simulink model "wind1000_BUS_Original"  (R2024a)
settings: Solver=ode45  StartTime=parSimulacio.ti  StopTime=40  MaxStep=parSimulacio.pas  RelTol=1e-6  AbsTol=1e-6  EnableMultiTasking=on
model workspace (MAT data in the model file):
  tt_vw : Simulink.Parameter (value not decoded)
values from neighbouring files (symbols this model uses):
  L = L'    [parameters_windFarm2.m]
  nu = size(S, 1)    [parameters_windFarm2.m]
undefined symbols (not defined in the file or in its folder): parControl, parConvertidor, parGenerador, parGrid, parMecanica, parSimulacio, S, w0, windspeed
(part 8 of 218)

== system wind1000_BUS_Original/Subsystem_200/Subsystem/String8/WT9 ==
  V_in : Inport
  I_in : Inport  Port=2
  Add1 : Sum  Inputs=+-
  C_cable : Subsystem
  C_filter : Subsystem
  Cable line : Subsystem
  Gain : Gain  Gain=1/parGrid.WTstring.Rparallel_cable
  Transformer-complex : Subsystem
  WFG : Subsystem
  V_out : Outport
  I_out : Outport  Port=2
  wires:
  I_in/1 -> C_cable/2(I_in)
  Gain/1 -> Add1/2
  Add1/1 -> C_cable/1(It2)
  Transformer-complex/2(It2) -> Add1/1
  V_in/1 -> Cable line/1(V)
  Transformer-complex/1(It1) -> C_filter/2(It1)
  Cable line/1(I_cable) -> C_cable/3(I_cable), I_out/1
  C_filter/1(Vc_filter_qd) -> Transformer-complex/1(Vc_filter_qd), WFG/1(vcqd)
  WFG/1(ilqd) -> C_filter/1(IWF)
  C_cable/1(Vc_cable) -> Cable line/2(Vc_cable), V_out/1, Transformer-complex/2(Vc_cable_qd), Gain/1

== system wind1000_BUS_Original/Subsystem_200/Subsystem/String9/WT1 ==
  V_in : Inport
  I_in : Inport  Port=2
  Add1 : Sum  Inputs=+-
  C_cable : Subsystem
  C_filter : Subsystem
  Cable line : Subsystem
  Gain : Gain  Gain=1/parGrid.WTstring.Rparallel_cable
  Transformer-complex : Subsystem
  WFG : Subsystem
  V_out : Outport
  I_out : Outport  Port=2
  wires:
  I_in/1 -> C_cable/2(I_in)
  Gain/1 -> Add1/2
  Add1/1 -> C_cable/1(It2)
  Transformer-complex/2(It2) -> Add1/1
  V_in/1 -> Cable line/1(V)
  Transformer-complex/1(It1) -> C_filter/2(It1)
  Cable line/1(I_cable) -> C_cable/3(I_cable), I_out/1
  C_filter/1(Vc_filter_qd) -> WFG/1(vcqd), Transformer-complex/1(Vc_filter_qd)
  WFG/1(ilqd) -> C_filter/1(IWF)
  C_cable/1(Vc_cable) -> Cable line/2(Vc_cable), V_out/1, Transformer-complex/2(Vc_cable_qd), Gain/1

== system wind1000_BUS_Original/Subsystem_200/Subsystem/String9/WT10 ==
  V_in : Inport
  I_in : Inport  Port=2
  Add1 : Sum  Inputs=+-
  C_cable : Subsystem
  C_filter : Subsystem
  Cable line : Subsystem
  Gain : Gain  Gain=1/parGrid.WTstring.Rparallel_cable
  Transformer-complex : Subsystem
  WFG : Subsystem
  V_out : Outport
  I_out : Outport  Port=2
  wires:
  C_cable/1(Vc_cable) -> Gain/1, Transformer-complex/2(Vc_cable_qd), V_out/1, Cable line/2(Vc_cable)
  WFG/1(ilqd) -> C_filter/1(IWF)
  C_filter/1(Vc_filter_qd) -> Transformer-complex/1(Vc_filter_qd), WFG/1(vcqd)
  Cable line/1(I_cable) -> I_out/1, C_cable/3(I_cable)
  Transformer-complex/1(It1) -> C_filter/2(It1)
  V_in/1 -> Cable line/1(V)
  Transformer-complex/2(It2) -> Add1/1
  Add1/1 -> C_cable/1(It2)
  Gain/1 -> Add1/2
  I_in/1 -> C_cable/2(I_in)

== system wind1000_BUS_Original/Subsystem_200/Subsystem/String9/WT2 ==
  V_in : Inport
  I_in : Inport  Port=2
  Add1 : Sum  Inputs=+-
  C_cable : Subsystem
  C_filter : Subsystem
  Cable line : Subsystem
  Gain : Gain  Gain=1/parGrid.WTstring.Rparallel_cable
  Transformer-complex : Subsystem
  WFG : Subsystem
  V_out : Outport
  I_out : Outport  Port=2
  wires:
  C_cable/1(Vc_cable) -> Gain/1, Transformer-complex/2(Vc_cable_qd), V_out/1, Cable line/2(Vc_cable)
  WFG/1(ilqd) -> C_filter/1(IWF)
  C_filter/1(Vc_filter_qd) -> Transformer-complex/1(Vc_filter_qd), WFG/1(vcqd)
  Cable line/1(I_cable) -> I_out/1, C_cable/3(I_cable)
  Transformer-complex/1(It1) -> C_filter/2(It1)
  V_in/1 -> Cable line/1(V)
  Transformer-complex/2(It2) -> Add1/1
  Add1/1 -> C_cable/1(It2)
  Gain/1 -> Add1/2
  I_in/1 -> C_cable/2(I_in)

== system wind1000_BUS_Original/Subsystem_200/Subsystem/String9/WT3 ==
  V_in : Inport
  I_in : Inport  Port=2
  Add1 : Sum  Inputs=+-
  C_cable : Subsystem
  C_filter : Subsystem
  Cable line : Subsystem
  Gain : Gain  Gain=1/parGrid.WTstring.Rparallel_cable
  Transformer-complex : Subsystem
  WFG : Subsystem
  V_out : Outport
  I_out : Outport  Port=2
  wires:
  I_in/1 -> C_cable/2(I_in)
  Gain/1 -> Add1/2
  Add1/1 -> C_cable/1(It2)
  Transformer-complex/2(It2) -> Add1/1
  V_in/1 -> Cable line/1(V)
  Transformer-complex/1(It1) -> C_filter/2(It1)
  Cable line/1(I_cable) -> C_cable/3(I_cable), I_out/1
  C_filter/1(Vc_filter_qd) -> Transformer-complex/1(Vc_filter_qd), WFG/1(vcqd)
  WFG/1(ilqd) -> C_filter/1(IWF)
  C_cable/1(Vc_cable) -> Cable line/2(Vc_cable), V_out/1, Transformer-complex/2(Vc_cable_qd), Gain/1

== system wind1000_BUS_Original/Subsystem_200/Subsystem/String9/WT4 ==
  V_in : Inport
  I_in : Inport  Port=2
  Add1 : Sum  Inputs=+-
  C_cable : Subsystem
  C_filter : Subsystem
  Cable line : Subsystem
  Gain : Gain  Gain=1/parGrid.WTstring.Rparallel_cable
  Transformer-complex : Subsystem
  WFG : Subsystem
  V_out : Outport
  I_out : Outport  Port=2
  wires:
  C_cable/1(Vc_cable) -> Gain/1, Transformer-complex/2(Vc_cable_qd), V_out/1, Cable line/2(Vc_cable)
  WFG/1(ilqd) -> C_filter/1(IWF)
  C_filter/1(Vc_filter_qd) -> Transformer-complex/1(Vc_filter_qd), WFG/1(vcqd)
  Cable line/1(I_cable) -> I_out/1, C_cable/3(I_cable)
  Transformer-complex/1(It1) -> C_filter/2(It1)
  V_in/1 -> Cable line/1(V)
  Transformer-complex/2(It2) -> Add1/1
  Add1/1 -> C_cable/1(It2)
  Gain/1 -> Add1/2
  I_in/1 -> C_cable/2(I_in)

== system wind1000_BUS_Original/Subsystem_200/Subsystem/String9/WT5 ==
  V_in : Inport
  I_in : Inport  Port=2
  Add1 : Sum  Inputs=+-
  C_cable : Subsystem
  C_filter : Subsystem
  Cable line : Subsystem
  Gain : Gain  Gain=1/parGrid.WTstring.Rparallel_cable
  Transformer-complex : Subsystem
  WFG : Subsystem
  V_out : Outport
  I_out : Outport  Port=2
  wires:
  I_in/1 -> C_cable/2(I_in)
  Gain/1 -> Add1/2
  Add1/1 -> C_cable/1(It2)
  Transformer-complex/2(It2) -> Add1/1
  V_in/1 -> Cable line/1(V)
  Transformer-complex/1(It1) -> C_filter/2(It1)
  Cable line/1(I_cable) -> C_cable/3(I_cable), I_out/1
  C_filter/1(Vc_filter_qd) -> Transformer-complex/1(Vc_filter_qd), WFG/1(vcqd)
  WFG/1(ilqd) -> C_filter/1(IWF)
  C_cable/1(Vc_cable) -> Cable line/2(Vc_cable), V_out/1, Transformer-complex/2(Vc_cable_qd), Gain/1

== system wind1000_BUS_Original/Subsystem_200/Subsystem/String9/WT6 ==
  V_in : Inport
  I_in : Inport  Port=2
  Add1 : Sum  Inputs=+-
  C_cable : Subsystem
  C_filter : Subsystem
  Cable line : Subsystem
  Gain : Gain  Gain=1/parGrid.WTstring.Rparallel_cable
  Transformer-complex : Subsystem
  WFG : Subsystem
  V_out : Outport
  I_out : Outport  Port=2
  wires:
  C_cable/1(Vc_cable) -> Gain/1, Transformer-complex/2(Vc_cable_qd), V_out/1, Cable line/2(Vc_cable)
  WFG/1(ilqd) -> C_filter/1(IWF)
  C_filter/1(Vc_filter_qd) -> Transformer-complex/1(Vc_filter_qd), WFG/1(vcqd)
  Cable line/1(I_cable) -> I_out/1, C_cable/3(I_cable)
  Transformer-complex/1(It1) -> C_filter/2(It1)
  V_in/1 -> Cable line/1(V)
  Transformer-complex/2(It2) -> Add1/1
  Add1/1 -> C_cable/1(It2)
  Gain/1 -> Add1/2
  I_in/1 -> C_cable/2(I_in)

== system wind1000_BUS_Original/Subsystem_200/Subsystem/String9/WT7 ==
  V_in : Inport
  I_in : Inport  Port=2
  Add1 : Sum  Inputs=+-
  C_cable : Subsystem
  C_filter : Subsystem
  Cable line : Subsystem
  Gain : Gain  Gain=1/parGrid.WTstring.Rparallel_cable
  Transformer-complex : Subsystem
  WFG : Subsystem
  V_out : Outport
  I_out : Outport  Port=2
  wires:
  I_in/1 -> C_cable/2(I_in)
  Gain/1 -> Add1/2
  Add1/1 -> C_cable/1(It2)
  Transformer-complex/2(It2) -> Add1/1
  V_in/1 -> Cable line/1(V)
  Transformer-complex/1(It1) -> C_filter/2(It1)
  Cable line/1(I_cable) -> C_cable/3(I_cable), I_out/1
  C_filter/1(Vc_filter_qd) -> Transformer-complex/1(Vc_filter_qd), WFG/1(vcqd)
  WFG/1(ilqd) -> C_filter/1(IWF)
  C_cable/1(Vc_cable) -> Cable line/2(Vc_cable), V_out/1, Transformer-complex/2(Vc_cable_qd), Gain/1

== system wind1000_BUS_Original/Subsystem_200/Subsystem/String9/WT8 ==
  V_in : Inport
  I_in : Inport  Port=2
  Add1 : Sum  Inputs=+-
  C_cable : Subsystem
  C_filter : Subsystem
  Cable line : Subsystem
  Gain : Gain  Gain=1/parGrid.WTstring.Rparallel_cable
  Transformer-complex : Subsystem
  WFG : Subsystem
  V_out : Outport
  I_out : Outport  Port=2
  wires:
  C_cable/1(Vc_cable) -> Gain/1, Transformer-complex/2(Vc_cable_qd), V_out/1, Cable line/2(Vc_cable)
  WFG/1(ilqd) -> C_filter/1(IWF)
  C_filter/1(Vc_filter_qd) -> Transformer-complex/1(Vc_filter_qd), WFG/1(vcqd)
  Cable line/1(I_cable) -> I_out/1, C_cable/3(I_cable)
  Transformer-complex/1(It1) -> C_filter/2(It1)
  V_in/1 -> Cable line/1(V)
  Transformer-complex/2(It2) -> Add1/1
  Add1/1 -> C_cable/1(It2)
  Gain/1 -> Add1/2
  I_in/1 -> C_cable/2(I_in)

== system wind1000_BUS_Original/Subsystem_200/Subsystem/String9/WT9 ==
  V_in : Inport
  I_in : Inport  Port=2
  Add1 : Sum  Inputs=+-
  C_cable : Subsystem
  C_filter : Subsystem
  Cable line : Subsystem
  Gain : Gain  Gain=1/parGrid.WTstring.Rparallel_cable
  Transformer-complex : Subsystem
  WFG : Subsystem
  V_out : Outport
  I_out : Outport  Port=2
  wires:
  I_in/1 -> C_cable/2(I_in)
  Gain/1 -> Add1/2
  Add1/1 -> C_cable/1(It2)
  Transformer-complex/2(It2) -> Add1/1
  V_in/1 -> Cable line/1(V)
  Transformer-complex/1(It1) -> C_filter/2(It1)
  Cable line/1(I_cable) -> C_cable/3(I_cable), I_out/1
  C_filter/1(Vc_filter_qd) -> Transformer-complex/1(Vc_filter_qd), WFG/1(vcqd)
  WFG/1(ilqd) -> C_filter/1(IWF)
  C_cable/1(Vc_cable) -> Cable line/2(Vc_cable), V_out/1, Transformer-complex/2(Vc_cable_qd), Gain/1

== system wind1000_BUS_Original/Subsystem/Subsystem_1/3phase2dq/Font trifasica asimetrica1/Park ==
  theta_r : Inport
  Constant4 : Constant  Value=2*pi/3
  Gain7 : Gain  Gain=2/3
  Matrix Concatenate1 : Concatenate  NumInputs=3  Mode=Multidimensional array  ConcatenateDimension=2
  Matrix Concatenate2 : Concatenate  Mode=Multidimensional array  ConcatenateDimension=2
  Mux2 : Mux  Inputs=2
  Mux3 : Mux  Inputs=2
  Mux4 : Mux  Inputs=2
  Mux5 : Mux  Inputs=3
  Mux6 : Mux  Inputs=3
  Sum1 : Sum  Inputs=|++
  Sum2 : Sum  Inputs=|+-
  Trigonometric Function : Trigonometry  Operator=cos
  Trigonometric Function1 : Trigonometry
  Trigonometric Function2 : Trigonometry  Operator=cos
  Trigonometric Function3 : Trigonometry
  Trigonometric Function4 : Trigonometry  Operator=cos
  Trigonometric Function5 : Trigonometry
  Tthetar : Outport
  nTthetar : Outport  Port=2
  wires:
  theta_r/1 -> Sum1/1, Sum2/1, Trigonometric Function/1, Trigonometric Function1/1
  Sum2/1 -> Trigonometric Function2/1, Trigonometric Function3/1
  Sum1/1 -> Trigonometric Function4/1, Trigonometric Function5/1
  Constant4/1 -> Sum2/2, Sum1/2
  Trigonometric Function/1 -> Mux2/1, Mux5/1
  Trigonometric Function1/1 -> Mux2/2, Mux6/1
  Trigonometric Function2/1 -> Mux3/1, Mux5/2
  Trigonometric Function3/1 -> Mux3/2, Mux6/2
  Trigonometric Function4/1 -> Mux4/1, Mux5/3
  Trigonometric Function5/1 -> Mux4/2, Mux6/3
  Mux2/1 -> Matrix Concatenate1/1
  Mux3/1 -> Matrix Concatenate1/2
  Mux4/1 -> Matrix Concatenate1/3
  Matrix Concatenate1/1 -> Gain7/1
  Mux5/1 -> Matrix Concatenate2/1
  Mux6/1 -> Matrix Concatenate2/2
  Gain7/1 -> Tthetar/1
  Matrix Concatenate2/1 -> nTthetar/1

== system wind1000_BUS_Original/Subsystem/Subsystem_1/Subsystem/String1/WT1 ==
  V_in : Inport
  I_in : Inport  Port=2
  Add1 : Sum  Inputs=+-
  C_cable : Subsystem
  C_filter : Subsystem
  Cable line : Subsystem
  Gain : Gain  Gain=1/parGrid.WTstring.Rparallel_cable
  Transformer-complex : Subsystem
  WFG : Subsystem
  V_out : Outport
  I_out : Outport  Port=2
  wires:
  C_cable/1(Vc_cable) -> Gain/1, Transformer-complex/2(Vc_cable_qd), V_out/1, Cable line/2(Vc_cable)
  WFG/1(ilqd) -> C_filter/1(IWF)
  C_filter/1(Vc_filter_qd) -> Transformer-complex/1(Vc_filter_qd), WFG/1(vcqd)
  Cable line/1(I_cable) -> I_out/1, C_cable/3(I_cable)
  Transformer-complex/1(It1) -> C_filter/2(It1)
  V_in/1 -> Cable line/1(V)
  Transformer-complex/2(It2) -> Add1/1
  Add1/1 -> C_cable/1(It2)
  Gain/1 -> Add1/2
  I_in/1 -> C_cable/2(I_in)

== system wind1000_BUS_Original/Subsystem/Subsystem_1/Subsystem/String1/WT10 ==
  V_in : Inport
  I_in : Inport  Port=2
  Add1 : Sum  Inputs=+-
  C_cable : Subsystem
  C_filter : Subsystem
  Cable line : Subsystem
  Gain : Gain  Gain=1/parGrid.WTstring.Rparallel_cable
  Transformer-complex : Subsystem
  WFG : Subsystem
  V_out : Outport
  I_out : Outport  Port=2
  wires:
  I_in/1 -> C_cable/2(I_in)
  Gain/1 -> Add1/2
  Add1/1 -> C_cable/1(It2)
  Transformer-complex/2(It2) -> Add1/1
  V_in/1 -> Cable line/1(V)
  Transformer-complex/1(It1) -> C_filter/2(It1)
  Cable line/1(I_cable) -> C_cable/3(I_cable), I_out/1
  C_filter/1(Vc_filter_qd) -> WFG/1(vcqd), Transformer-complex/1(Vc_filter_qd)
  WFG/1(ilqd) -> C_filter/1(IWF)
  C_cable/1(Vc_cable) -> Cable line/2(Vc_cable), V_out/1, Transformer-complex/2(Vc_cable_qd), Gain/1

== system wind1000_BUS_Original/Subsystem/Subsystem_1/Subsystem/String1/WT2 ==
  V_in : Inport
  I_in : Inport  Port=2
  Add1 : Sum  Inputs=+-
  C_cable : Subsystem
  C_filter : Subsystem
  Cable line : Subsystem
  Gain : Gain  Gain=1/parGrid.WTstring.Rparallel_cable
  Transformer-complex : Subsystem
  WFG : Subsystem
  V_out : Outport
  I_out : Outport  Port=2
  wires:
  I_in/1 -> C_cable/2(I_in)
  Gain/1 -> Add1/2
  Add1/1 -> C_cable/1(It2)
  Transformer-complex/2(It2) -> Add1/1
  V_in/1 -> Cable line/1(V)
  Transformer-complex/1(It1) -> C_filter/2(It1)
  Cable line/1(I_cable) -> C_cable/3(I_cable), I_out/1
  C_filter/1(Vc_filter_qd) -> WFG/1(vcqd), Transformer-complex/1(Vc_filter_qd)
  WFG/1(ilqd) -> C_filter/1(IWF)
  C_cable/1(Vc_cable) -> Cable line/2(Vc_cable), V_out/1, Transformer-complex/2(Vc_cable_qd), Gain/1

== system wind1000_BUS_Original/Subsystem/Subsystem_1/Subsystem/String1/WT3 ==
  V_in : Inport
  I_in : Inport  Port=2
  Add1 : Sum  Inputs=+-
  C_cable : Subsystem
  C_filter : Subsystem
  Cable line : Subsystem
  Gain : Gain  Gain=1/parGrid.WTstring.Rparallel_cable
  Transformer-complex : Subsystem
  WFG : Subsystem
  V_out : Outport
  I_out : Outport  Port=2
  wires:
  C_cable/1(Vc_cable) -> Gain/1, Transformer-complex/2(Vc_cable_qd), V_out/1, Cable line/2(Vc_cable)
  WFG/1(ilqd) -> C_filter/1(IWF)
  C_filter/1(Vc_filter_qd) -> WFG/1(vcqd), Transformer-complex/1(Vc_filter_qd)
  Cable line/1(I_cable) -> I_out/1, C_cable/3(I_cable)
  Transformer-complex/1(It1) -> C_filter/2(It1)
  V_in/1 -> Cable line/1(V)
  Transformer-complex/2(It2) -> Add1/1
  Add1/1 -> C_cable/1(It2)
  Gain/1 -> Add1/2
  I_in/1 -> C_cable/2(I_in)

== system wind1000_BUS_Original/Subsystem/Subsystem_1/Subsystem/String1/WT4 ==
  V_in : Inport
  I_in : Inport  Port=2
  Add1 : Sum  Inputs=+-
  C_cable : Subsystem
  C_filter : Subsystem
  Cable line : Subsystem
  Gain : Gain  Gain=1/parGrid.WTstring.Rparallel_cable
  Transformer-complex : Subsystem
  WFG : Subsystem
  V_out : Outport
  I_out : Outport  Port=2
  wires:
  I_in/1 -> C_cable/2(I_in)
  Gain/1 -> Add1/2
  Add1/1 -> C_cable/1(It2)
  Transformer-complex/2(It2) -> Add1/1
  V_in/1 -> Cable line/1(V)
  Transformer-complex/1(It1) -> C_filter/2(It1)
  Cable line/1(I_cable) -> C_cable/3(I_cable), I_out/1
  C_filter/1(Vc_filter_qd) -> WFG/1(vcqd), Transformer-complex/1(Vc_filter_qd)
  WFG/1(ilqd) -> C_filter/1(IWF)
  C_cable/1(Vc_cable) -> Cable line/2(Vc_cable), V_out/1, Transformer-complex/2(Vc_cable_qd), Gain/1

== system wind1000_BUS_Original/Subsystem/Subsystem_1/Subsystem/String1/WT5 ==
  V_in : Inport
  I_in : Inport  Port=2
  Add1 : Sum  Inputs=+-
  C_cable : Subsystem
  C_filter : Subsystem
  Cable line : Subsystem
  Gain : Gain  Gain=1/parGrid.WTstring.Rparallel_cable
  Transformer-complex : Subsystem
  WFG : Subsystem
  V_out : Outport
  I_out : Outport  Port=2
  wires:
  C_cable/1(Vc_cable) -> Gain/1, Transformer-complex/2(Vc_cable_qd), V_out/1, Cable line/2(Vc_cable)
  WFG/1(ilqd) -> C_filter/1(IWF)
  C_filter/1(Vc_filter_qd) -> WFG/1(vcqd), Transformer-complex/1(Vc_filter_qd)
  Cable line/1(I_cable) -> I_out/1, C_cable/3(I_cable)
  Transformer-complex/1(It1) -> C_filter/2(It1)
  V_in/1 -> Cable line/1(V)
  Transformer-complex/2(It2) -> Add1/1
  Add1/1 -> C_cable/1(It2)
  Gain/1 -> Add1/2
  I_in/1 -> C_cable/2(I_in)

== system wind1000_BUS_Original/Subsystem/Subsystem_1/Subsystem/String1/WT6 ==
  V_in : Inport
  I_in : Inport  Port=2
  Add1 : Sum  Inputs=+-
  C_cable : Subsystem
  C_filter : Subsystem
  Cable line : Subsystem
  Gain : Gain  Gain=1/parGrid.WTstring.Rparallel_cable
  Transformer-complex : Subsystem
  WFG : Subsystem
  V_out : Outport
  I_out : Outport  Port=2
  wires:
  I_in/1 -> C_cable/2(I_in)
  Gain/1 -> Add1/2
  Add1/1 -> C_cable/1(It2)
  Transformer-complex/2(It2) -> Add1/1
  V_in/1 -> Cable line/1(V)
  Transformer-complex/1(It1) -> C_filter/2(It1)
  Cable line/1(I_cable) -> C_cable/3(I_cable), I_out/1
  C_filter/1(Vc_filter_qd) -> WFG/1(vcqd), Transformer-complex/1(Vc_filter_qd)
  WFG/1(ilqd) -> C_filter/1(IWF)
  C_cable/1(Vc_cable) -> Cable line/2(Vc_cable), V_out/1, Transformer-complex/2(Vc_cable_qd), Gain/1

== system wind1000_BUS_Original/Subsystem/Subsystem_1/Subsystem/String1/WT7 ==
  V_in : Inport
  I_in : Inport  Port=2
  Add1 : Sum  Inputs=+-
  C_cable : Subsystem
  C_filter : Subsystem
  Cable line : Subsystem
  Gain : Gain  Gain=1/parGrid.WTstring.Rparallel_cable
  Transformer-complex : Subsystem
  WFG : Subsystem
  V_out : Outport
  I_out : Outport  Port=2
  wires:
  C_cable/1(Vc_cable) -> Gain/1, Transformer-complex/2(Vc_cable_qd), V_out/1, Cable line/2(Vc_cable)
  WFG/1(ilqd) -> C_filter/1(IWF)
  C_filter/1(Vc_filter_qd) -> WFG/1(vcqd), Transformer-complex/1(Vc_filter_qd)
  Cable line/1(I_cable) -> I_out/1, C_cable/3(I_cable)
  Transformer-complex/1(It1) -> C_filter/2(It1)
  V_in/1 -> Cable line/1(V)
  Transformer-complex/2(It2) -> Add1/1
  Add1/1 -> C_cable/1(It2)
  Gain/1 -> Add1/2
  I_in/1 -> C_cable/2(I_in)

== system wind1000_BUS_Original/Subsystem/Subsystem_1/Subsystem/String1/WT8 ==
  V_in : Inport
  I_in : Inport  Port=2
  Add1 : Sum  Inputs=+-
  C_cable : Subsystem
  C_filter : Subsystem
  Cable line : Subsystem
  Gain : Gain  Gain=1/parGrid.WTstring.Rparallel_cable
  Transformer-complex : Subsystem
  WFG : Subsystem
  V_out : Outport
  I_out : Outport  Port=2
  wires:
  I_in/1 -> C_cable/2(I_in)
  Gain/1 -> Add1/2
  Add1/1 -> C_cable/1(It2)
  Transformer-complex/2(It2) -> Add1/1
  V_in/1 -> Cable line/1(V)
  Transformer-complex/1(It1) -> C_filter/2(It1)
  Cable line/1(I_cable) -> C_cable/3(I_cable), I_out/1
  C_filter/1(Vc_filter_qd) -> WFG/1(vcqd), Transformer-complex/1(Vc_filter_qd)
  WFG/1(ilqd) -> C_filter/1(IWF)
  C_cable/1(Vc_cable) -> Cable line/2(Vc_cable), V_out/1, Transformer-complex/2(Vc_cable_qd), Gain/1

== system wind1000_BUS_Original/Subsystem/Subsystem_1/Subsystem/String1/WT9 ==
  V_in : Inport
  I_in : Inport  Port=2
  Add1 : Sum  Inputs=+-
  C_cable : Subsystem
  C_filter : Subsystem
  Cable line : Subsystem
  Gain : Gain  Gain=1/parGrid.WTstring.Rparallel_cable
  Transformer-complex : Subsystem
  WFG : Subsystem
  V_out : Outport
  I_out : Outport  Port=2
  wires:
  C_cable/1(Vc_cable) -> Gain/1, Transformer-complex/2(Vc_cable_qd), V_out/1, Cable line/2(Vc_cable)
  WFG/1(ilqd) -> C_filter/1(IWF)
  C_filter/1(Vc_filter_qd) -> WFG/1(vcqd), Transformer-complex/1(Vc_filter_qd)
  Cable line/1(I_cable) -> I_out/1, C_cable/3(I_cable)
  Transformer-complex/1(It1) -> C_filter/2(It1)
  V_in/1 -> Cable line/1(V)
  Transformer-complex/2(It2) -> Add1/1
  Add1/1 -> C_cable/1(It2)
  Gain/1 -> Add1/2
  I_in/1 -> C_cable/2(I_in)

== system wind1000_BUS_Original/Subsystem/Subsystem_1/Subsystem/String2/WT1 ==
  V_in : Inport
  I_in : Inport  Port=2
  Add1 : Sum  Inputs=+-
  C_cable : Subsystem
  C_filter : Subsystem
  Cable line : Subsystem
  Gain : Gain  Gain=1/parGrid.WTstring.Rparallel_cable
  Transformer-complex : Subsystem
  WFG : Subsystem
  V_out : Outport
  I_out : Outport  Port=2
  wires:
  I_in/1 -> C_cable/2(I_in)
  Gain/1 -> Add1/2
  Add1/1 -> C_cable/1(It2)
  Transformer-complex/2(It2) -> Add1/1
  V_in/1 -> Cable line/1(V)
  Transformer-complex/1(It1) -> C_filter/2(It1)
  Cable line/1(I_cable) -> C_cable/3(I_cable), I_out/1
  C_filter/1(Vc_filter_qd) -> WFG/1(vcqd), Transformer-complex/1(Vc_filter_qd)
  WFG/1(ilqd) -> C_filter/1(IWF)
  C_cable/1(Vc_cable) -> Cable line/2(Vc_cable), V_out/1, Transformer-complex/2(Vc_cable_qd), Gain/1

== system wind1000_BUS_Original/Subsystem/Subsystem_1/Subsystem/String2/WT10 ==
  V_in : Inport
  I_in : Inport  Port=2
  Add1 : Sum  Inputs=+-
  C_cable : Subsystem
  C_filter : Subsystem
  Cable line : Subsystem
  Gain : Gain  Gain=1/parGrid.WTstring.Rparallel_cable
  Transformer-complex : Subsystem
  WFG : Subsystem
  V_out : Outport
  I_out : Outport  Port=2
  wires:
  C_cable/1(Vc_cable) -> Gain/1, Transformer-complex/2(Vc_cable_qd), V_out/1, Cable line/2(Vc_cable)
  WFG/1(ilqd) -> C_filter/1(IWF)
  C_filter/1(Vc_filter_qd) -> Transformer-complex/1(Vc_filter_qd), WFG/1(vcqd)
  Cable line/1(I_cable) -> I_out/1, C_cable/3(I_cable)
  Transformer-complex/1(It1) -> C_filter/2(It1)
  V_in/1 -> Cable line/1(V)
  Transformer-complex/2(It2) -> Add1/1
  Add1/1 -> C_cable/1(It2)
  Gain/1 -> Add1/2
  I_in/1 -> C_cable/2(I_in)

== system wind1000_BUS_Original/Subsystem/Subsystem_1/Subsystem/String2/WT2 ==
  V_in : Inport
  I_in : Inport  Port=2
  Add1 : Sum  Inputs=+-
  C_cable : Subsystem
  C_filter : Subsystem
  Cable line : Subsystem
  Gain : Gain  Gain=1/parGrid.WTstring.Rparallel_cable
  Transformer-complex : Subsystem
  WFG : Subsystem
  V_out : Outport
  I_out : Outport  Port=2
  wires:
  C_cable/1(Vc_cable) -> Gain/1, Transformer-complex/2(Vc_cable_qd), V_out/1, Cable line/2(Vc_cable)
  WFG/1(ilqd) -> C_filter/1(IWF)
  C_filter/1(Vc_filter_qd) -> Transformer-complex/1(Vc_filter_qd), WFG/1(vcqd)
  Cable line/1(I_cable) -> I_out/1, C_cable/3(I_cable)
  Transformer-complex/1(It1) -> C_filter/2(It1)
  V_in/1 -> Cable line/1(V)
  Transformer-complex/2(It2) -> Add1/1
  Add1/1 -> C_cable/1(It2)
  Gain/1 -> Add1/2
  I_in/1 -> C_cable/2(I_in)

== system wind1000_BUS_Original/Subsystem/Subsystem_1/Subsystem/String2/WT3 ==
  V_in : Inport
  I_in : Inport  Port=2
  Add1 : Sum  Inputs=+-
  C_cable : Subsystem
  C_filter : Subsystem
  Cable line : Subsystem
  Gain : Gain  Gain=1/parGrid.WTstring.Rparallel_cable
  Transformer-complex : Subsystem
  WFG : Subsystem
  V_out : Outport
  I_out : Outport  Port=2
  wires:
  I_in/1 -> C_cable/2(I_in)
  Gain/1 -> Add1/2
  Add1/1 -> C_cable/1(It2)
  Transformer-complex/2(It2) -> Add1/1
  V_in/1 -> Cable line/1(V)
  Transformer-complex/1(It1) -> C_filter/2(It1)
  Cable line/1(I_cable) -> C_cable/3(I_cable), I_out/1
  C_filter/1(Vc_filter_qd) -> Transformer-complex/1(Vc_filter_qd), WFG/1(vcqd)
  WFG/1(ilqd) -> C_filter/1(IWF)
  C_cable/1(Vc_cable) -> Cable line/2(Vc_cable), V_out/1, Transformer-complex/2(Vc_cable_qd), Gain/1

== system wind1000_BUS_Original/Subsystem/Subsystem_1/Subsystem/String2/WT4 ==
  V_in : Inport
  I_in : Inport  Port=2
  Add1 : Sum  Inputs=+-
  C_cable : Subsystem
  C_filter : Subsystem
  Cable line : Subsystem
  Gain : Gain  Gain=1/parGrid.WTstring.Rparallel_cable
  Transformer-complex : Subsystem
  WFG : Subsystem
  V_out : Outport
  I_out : Outport  Port=2
  wires:
  C_cable/1(Vc_cable) -> Gain/1, Transformer-complex/2(Vc_cable_qd), V_out/1, Cable line/2(Vc_cable)
  WFG/1(ilqd) -> C_filter/1(IWF)
  C_filter/1(Vc_filter_qd) -> Transformer-complex/1(Vc_filter_qd), WFG/1(vcqd)
  Cable line/1(I_cable) -> I_out/1, C_cable/3(I_cable)
  Transformer-complex/1(It1) -> C_filter/2(It1)
  V_in/1 -> Cable line/1(V)
  Transformer-complex/2(It2) -> Add1/1
  Add1/1 -> C_cable/1(It2)
  Gain/1 -> Add1/2
  I_in/1 -> C_cable/2(I_in)

== system wind1000_BUS_Original/Subsystem/Subsystem_1/Subsystem/String2/WT5 ==
  V_in : Inport
  I_in : Inport  Port=2
  Add1 : Sum  Inputs=+-
  C_cable : Subsystem
  C_filter : Subsystem
  Cable line : Subsystem
  Gain : Gain  Gain=1/parGrid.WTstring.Rparallel_cable
  Transformer-complex : Subsystem
  WFG : Subsystem
  V_out : Outport
  I_out : Outport  Port=2
  wires:
  I_in/1 -> C_cable/2(I_in)
  Gain/1 -> Add1/2
  Add1/1 -> C_cable/1(It2)
  Transformer-complex/2(It2) -> Add1/1
  V_in/1 -> Cable line/1(V)
  Transformer-complex/1(It1) -> C_filter/2(It1)
  Cable line/1(I_cable) -> C_cable/3(I_cable), I_out/1
  C_filter/1(Vc_filter_qd) -> Transformer-complex/1(Vc_filter_qd), WFG/1(vcqd)
  WFG/1(ilqd) -> C_filter/1(IWF)
  C_cable/1(Vc_cable) -> Cable line/2(Vc_cable), V_out/1, Transformer-complex/2(Vc_cable_qd), Gain/1

== system wind1000_BUS_Original/Subsystem/Subsystem_1/Subsystem/String2/WT6 ==
  V_in : Inport
  I_in : Inport  Port=2
  Add1 : Sum  Inputs=+-
  C_cable : Subsystem
  C_filter : Subsystem
  Cable line : Subsystem
  Gain : Gain  Gain=1/parGrid.WTstring.Rparallel_cable
  Transformer-complex : Subsystem
  WFG : Subsystem
  V_out : Outport
  I_out : Outport  Port=2
  wires:
  C_cable/1(Vc_cable) -> Gain/1, Transformer-complex/2(Vc_cable_qd), V_out/1, Cable line/2(Vc_cable)
  WFG/1(ilqd) -> C_filter/1(IWF)
  C_filter/1(Vc_filter_qd) -> Transformer-complex/1(Vc_filter_qd), WFG/1(vcqd)
  Cable line/1(I_cable) -> I_out/1, C_cable/3(I_cable)
  Transformer-complex/1(It1) -> C_filter/2(It1)
  V_in/1 -> Cable line/1(V)
  Transformer-complex/2(It2) -> Add1/1
  Add1/1 -> C_cable/1(It2)
  Gain/1 -> Add1/2
  I_in/1 -> C_cable/2(I_in)

== system wind1000_BUS_Original/Subsystem/Subsystem_1/Subsystem/String2/WT7 ==
  V_in : Inport
  I_in : Inport  Port=2
  Add1 : Sum  Inputs=+-
  C_cable : Subsystem
  C_filter : Subsystem
  Cable line : Subsystem
  Gain : Gain  Gain=1/parGrid.WTstring.Rparallel_cable
  Transformer-complex : Subsystem
  WFG : Subsystem
  V_out : Outport
  I_out : Outport  Port=2
  wires:
  I_in/1 -> C_cable/2(I_in)
  Gain/1 -> Add1/2
  Add1/1 -> C_cable/1(It2)
  Transformer-complex/2(It2) -> Add1/1
  V_in/1 -> Cable line/1(V)
  Transformer-complex/1(It1) -> C_filter/2(It1)
  Cable line/1(I_cable) -> C_cable/3(I_cable), I_out/1
  C_filter/1(Vc_filter_qd) -> Transformer-complex/1(Vc_filter_qd), WFG/1(vcqd)
  WFG/1(ilqd) -> C_filter/1(IWF)
  C_cable/1(Vc_cable) -> Cable line/2(Vc_cable), V_out/1, Transformer-complex/2(Vc_cable_qd), Gain/1

== system wind1000_BUS_Original/Subsystem/Subsystem_1/Subsystem/String2/WT8 ==
  V_in : Inport
  I_in : Inport  Port=2
  Add1 : Sum  Inputs=+-
  C_cable : Subsystem
  C_filter : Subsystem
  Cable line : Subsystem
  Gain : Gain  Gain=1/parGrid.WTstring.Rparallel_cable
  Transformer-complex : Subsystem
  WFG : Subsystem
  V_out : Outport
  I_out : Outport  Port=2
  wires:
  C_cable/1(Vc_cable) -> Gain/1, Transformer-complex/2(Vc_cable_qd), V_out/1, Cable line/2(Vc_cable)
  WFG/1(ilqd) -> C_filter/1(IWF)
  C_filter/1(Vc_filter_qd) -> Transformer-complex/1(Vc_filter_qd), WFG/1(vcqd)
  Cable line/1(I_cable) -> I_out/1, C_cable/3(I_cable)
  Transformer-complex/1(It1) -> C_filter/2(It1)
  V_in/1 -> Cable line/1(V)
  Transformer-complex/2(It2) -> Add1/1
  Add1/1 -> C_cable/1(It2)
  Gain/1 -> Add1/2
  I_in/1 -> C_cable/2(I_in)

== system wind1000_BUS_Original/Subsystem/Subsystem_1/Subsystem/String2/WT9 ==
  V_in : Inport
  I_in : Inport  Port=2
  Add1 : Sum  Inputs=+-
  C_cable : Subsystem
  C_filter : Subsystem
  Cable line : Subsystem
  Gain : Gain  Gain=1/parGrid.WTstring.Rparallel_cable
  Transformer-complex : Subsystem
  WFG : Subsystem
  V_out : Outport
  I_out : Outport  Port=2
  wires:
  I_in/1 -> C_cable/2(I_in)
  Gain/1 -> Add1/2
  Add1/1 -> C_cable/1(It2)
  Transformer-complex/2(It2) -> Add1/1
  V_in/1 -> Cable line/1(V)
  Transformer-complex/1(It1) -> C_filter/2(It1)
  Cable line/1(I_cable) -> C_cable/3(I_cable), I_out/1
  C_filter/1(Vc_filter_qd) -> Transformer-complex/1(Vc_filter_qd), WFG/1(vcqd)
  WFG/1(ilqd) -> C_filter/1(IWF)
  C_cable/1(Vc_cable) -> Cable line/2(Vc_cable), V_out/1, Transformer-complex/2(Vc_cable_qd), Gain/1

== system wind1000_BUS_Original/Subsystem/Subsystem_1/Subsystem/String3/WT1 ==
  V_in : Inport
  I_in : Inport  Port=2
  Add1 : Sum  Inputs=+-
  C_cable : Subsystem
  C_filter : Subsystem
  Cable line : Subsystem
  Gain : Gain  Gain=1/parGrid.WTstring.Rparallel_cable
  Transformer-complex : Subsystem
  WFG : Subsystem
  V_out : Outport
  I_out : Outport  Port=2
  wires:
  I_in/1 -> C_cable/2(I_in)
  Gain/1 -> Add1/2
  Add1/1 -> C_cable/1(It2)
  Transformer-complex/2(It2) -> Add1/1
  V_in/1 -> Cable line/1(V)
  Transformer-complex/1(It1) -> C_filter/2(It1)
  Cable line/1(I_cable) -> C_cable/3(I_cable), I_out/1
  C_filter/1(Vc_filter_qd) -> WFG/1(vcqd), Transformer-complex/1(Vc_filter_qd)
  WFG/1(ilqd) -> C_filter/1(IWF)
  C_cable/1(Vc_cable) -> Cable line/2(Vc_cable), V_out/1, Transformer-complex/2(Vc_cable_qd), Gain/1

== system wind1000_BUS_Original/Subsystem/Subsystem_1/Subsystem/String3/WT10 ==
  V_in : Inport
  I_in : Inport  Port=2
  Add1 : Sum  Inputs=+-
  C_cable : Subsystem
  C_filter : Subsystem
  Cable line : Subsystem
  Gain : Gain  Gain=1/parGrid.WTstring.Rparallel_cable
  Transformer-complex : Subsystem
  WFG : Subsystem
  V_out : Outport
  I_out : Outport  Port=2
  wires:
  C_cable/1(Vc_cable) -> Gain/1, Transformer-complex/2(Vc_cable_qd), V_out/1, Cable line/2(Vc_cable)
  WFG/1(ilqd) -> C_filter/1(IWF)
  C_filter/1(Vc_filter_qd) -> Transformer-complex/1(Vc_filter_qd), WFG/1(vcqd)
  Cable line/1(I_cable) -> I_out/1, C_cable/3(I_cable)
  Transformer-complex/1(It1) -> C_filter/2(It1)
  V_in/1 -> Cable line/1(V)
  Transformer-complex/2(It2) -> Add1/1
  Add1/1 -> C_cable/1(It2)
  Gain/1 -> Add1/2
  I_in/1 -> C_cable/2(I_in)

== system wind1000_BUS_Original/Subsystem/Subsystem_1/Subsystem/String3/WT2 ==
  V_in : Inport
  I_in : Inport  Port=2
  Add1 : Sum  Inputs=+-
  C_cable : Subsystem
  C_filter : Subsystem
  Cable line : Subsystem
  Gain : Gain  Gain=1/parGrid.WTstring.Rparallel_cable
  Transformer-complex : Subsystem
  WFG : Subsystem
  V_out : Outport
  I_out : Outport  Port=2
  wires:
  C_cable/1(Vc_cable) -> Gain/1, Transformer-complex/2(Vc_cable_qd), V_out/1, Cable line/2(Vc_cable)
  WFG/1(ilqd) -> C_filter/1(IWF)
  C_filter/1(Vc_filter_qd) -> Transformer-complex/1(Vc_filter_qd), WFG/1(vcqd)
  Cable line/1(I_cable) -> I_out/1, C_cable/3(I_cable)
  Transformer-complex/1(It1) -> C_filter/2(It1)
  V_in/1 -> Cable line/1(V)
  Transformer-complex/2(It2) -> Add1/1
  Add1/1 -> C_cable/1(It2)
  Gain/1 -> Add1/2
  I_in/1 -> C_cable/2(I_in)

== system wind1000_BUS_Original/Subsystem/Subsystem_1/Subsystem/String3/WT3 ==
  V_in : Inport
  I_in : Inport  Port=2
  Add1 : Sum  Inputs=+-
  C_cable : Subsystem
  C_filter : Subsystem
  Cable line : Subsystem
  Gain : Gain  Gain=1/parGrid.WTstring.Rparallel_cable
  Transformer-complex : Subsystem
  WFG : Subsystem
  V_out : Outport
  I_out : Outport  Port=2
  wires:
  I_in/1 -> C_cable/2(I_in)
  Gain/1 -> Add1/2
  Add1/1 -> C_cable/1(It2)
  Transformer-complex/2(It2) -> Add1/1
  V_in/1 -> Cable line/1(V)
  Transformer-complex/1(It1) -> C_filter/2(It1)
  Cable line/1(I_cable) -> C_cable/3(I_cable), I_out/1
  C_filter/1(Vc_filter_qd) -> Transformer-complex/1(Vc_filter_qd), WFG/1(vcqd)
  WFG/1(ilqd) -> C_filter/1(IWF)
  C_cable/1(Vc_cable) -> Cable line/2(Vc_cable), V_out/1, Transformer-complex/2(Vc_cable_qd), Gain/1

== system wind1000_BUS_Original/Subsystem/Subsystem_1/Subsystem/String3/WT4 ==
  V_in : Inport
  I_in : Inport  Port=2
  Add1 : Sum  Inputs=+-
  C_cable : Subsystem
  C_filter : Subsystem
  Cable line : Subsystem
  Gain : Gain  Gain=1/parGrid.WTstring.Rparallel_cable
  Transformer-complex : Subsystem
  WFG : Subsystem
  V_out : Outport
  I_out : Outport  Port=2
  wires:
  C_cable/1(Vc_cable) -> Gain/1, Transformer-complex/2(Vc_cable_qd), V_out/1, Cable line/2(Vc_cable)
  WFG/1(ilqd) -> C_filter/1(IWF)
  C_filter/1(Vc_filter_qd) -> Transformer-complex/1(Vc_filter_qd), WFG/1(vcqd)
  Cable line/1(I_cable) -> I_out/1, C_cable/3(I_cable)
  Transformer-complex/1(It1) -> C_filter/2(It1)
  V_in/1 -> Cable line/1(V)
  Transformer-complex/2(It2) -> Add1/1
  Add1/1 -> C_cable/1(It2)
  Gain/1 -> Add1/2
  I_in/1 -> C_cable/2(I_in)

== system wind1000_BUS_Original/Subsystem/Subsystem_1/Subsystem/String3/WT5 ==
  V_in : Inport
  I_in : Inport  Port=2
  Add1 : Sum  Inputs=+-
  C_cable : Subsystem
  C_filter : Subsystem
  Cable line : Subsystem
  Gain : Gain  Gain=1/parGrid.WTstring.Rparallel_cable
  Transformer-complex : Subsystem
  WFG : Subsystem
  V_out : Outport
  I_out : Outport  Port=2
  wires:
  I_in/1 -> C_cable/2(I_in)
  Gain/1 -> Add1/2
  Add1/1 -> C_cable/1(It2)
  Transformer-complex/2(It2) -> Add1/1
  V_in/1 -> Cable line/1(V)
  Transformer-complex/1(It1) -> C_filter/2(It1)
  Cable line/1(I_cable) -> C_cable/3(I_cable), I_out/1
  C_filter/1(Vc_filter_qd) -> Transformer-complex/1(Vc_filter_qd), WFG/1(vcqd)
  WFG/1(ilqd) -> C_filter/1(IWF)
  C_cable/1(Vc_cable) -> Cable line/2(Vc_cable), V_out/1, Transformer-complex/2(Vc_cable_qd), Gain/1

== system wind1000_BUS_Original/Subsystem/Subsystem_1/Subsystem/String3/WT6 ==
  V_in : Inport
  I_in : Inport  Port=2
  Add1 : Sum  Inputs=+-
  C_cable : Subsystem
  C_filter : Subsystem
  Cable line : Subsystem
  Gain : Gain  Gain=1/parGrid.WTstring.Rparallel_cable
  Transformer-complex : Subsystem
  WFG : Subsystem
  V_out : Outport
  I_out : Outport  Port=2
  wires:
  C_cable/1(Vc_cable) -> Gain/1, Transformer-complex/2(Vc_cable_qd), V_out/1, Cable line/2(Vc_cable)
  WFG/1(ilqd) -> C_filter/1(IWF)
  C_filter/1(Vc_filter_qd) -> Transformer-complex/1(Vc_filter_qd), WFG/1(vcqd)
  Cable line/1(I_cable) -> I_out/1, C_cable/3(I_cable)
  Transformer-complex/1(It1) -> C_filter/2(It1)
  V_in/1 -> Cable line/1(V)
  Transformer-complex/2(It2) -> Add1/1
  Add1/1 -> C_cable/1(It2)
  Gain/1 -> Add1/2
  I_in/1 -> C_cable/2(I_in)

== system wind1000_BUS_Original/Subsystem/Subsystem_1/Subsystem/String3/WT7 ==
  V_in : Inport
  I_in : Inport  Port=2
  Add1 : Sum  Inputs=+-
  C_cable : Subsystem
  C_filter : Subsystem
  Cable line : Subsystem
  Gain : Gain  Gain=1/parGrid.WTstring.Rparallel_cable
  Transformer-complex : Subsystem
  WFG : Subsystem
  V_out : Outport
  I_out : Outport  Port=2
  wires:
  I_in/1 -> C_cable/2(I_in)
  Gain/1 -> Add1/2
  Add1/1 -> C_cable/1(It2)
  Transformer-complex/2(It2) -> Add1/1
  V_in/1 -> Cable line/1(V)
  Transformer-complex/1(It1) -> C_filter/2(It1)
  Cable line/1(I_cable) -> C_cable/3(I_cable), I_out/1
  C_filter/1(Vc_filter_qd) -> Transformer-complex/1(Vc_filter_qd), WFG/1(vcqd)
  WFG/1(ilqd) -> C_filter/1(IWF)
  C_cable/1(Vc_cable) -> Cable line/2(Vc_cable), V_out/1, Transformer-complex/2(Vc_cable_qd), Gain/1

== system wind1000_BUS_Original/Subsystem/Subsystem_1/Subsystem/String3/WT8 ==
  V_in : Inport
  I_in : Inport  Port=2
  Add1 : Sum  Inputs=+-
  C_cable : Subsystem
  C_filter : Subsystem
  Cable line : Subsystem
  Gain : Gain  Gain=1/parGrid.WTstring.Rparallel_cable
  Transformer-complex : Subsystem
  WFG : Subsystem
  V_out : Outport
  I_out : Outport  Port=2
  wires:
  C_cable/1(Vc_cable) -> Gain/1, Transformer-complex/2(Vc_cable_qd), V_out/1, Cable line/2(Vc_cable)
  WFG/1(ilqd) -> C_filter/1(IWF)
  C_filter/1(Vc_filter_qd) -> Transformer-complex/1(Vc_filter_qd), WFG/1(vcqd)
  Cable line/1(I_cable) -> I_out/1, C_cable/3(I_cable)
  Transformer-complex/1(It1) -> C_filter/2(It1)
  V_in/1 -> Cable line/1(V)
  Transformer-complex/2(It2) -> Add1/1
  Add1/1 -> C_cable/1(It2)
  Gain/1 -> Add1/2
  I_in/1 -> C_cable/2(I_in)

== system wind1000_BUS_Original/Subsystem/Subsystem_1/Subsystem/String3/WT9 ==
  V_in : Inport
  I_in : Inport  Port=2
  Add1 : Sum  Inputs=+-
  C_cable : Subsystem
  C_filter : Subsystem
  Cable line : Subsystem
  Gain : Gain  Gain=1/parGrid.WTstring.Rparallel_cable
  Transformer-complex : Subsystem
  WFG : Subsystem
  V_out : Outport
  I_out : Outport  Port=2
  wires:
  I_in/1 -> C_cable/2(I_in)
  Gain/1 -> Add1/2
  Add1/1 -> C_cable/1(It2)
  Transformer-complex/2(It2) -> Add1/1
  V_in/1 -> Cable line/1(V)
  Transformer-complex/1(It1) -> C_filter/2(It1)
  Cable line/1(I_cable) -> C_cable/3(I_cable), I_out/1
  C_filter/1(Vc_filter_qd) -> Transformer-complex/1(Vc_filter_qd), WFG/1(vcqd)
  WFG/1(ilqd) -> C_filter/1(IWF)
  C_cable/1(Vc_cable) -> Cable line/2(Vc_cable), V_out/1, Transformer-complex/2(Vc_cable_qd), Gain/1

== system wind1000_BUS_Original/Subsystem/Subsystem_1/Subsystem/String4/WT1 ==
  V_in : Inport
  I_in : Inport  Port=2
  Add1 : Sum  Inputs=+-
  C_cable : Subsystem
  C_filter : Subsystem
  Cable line : Subsystem
  Gain : Gain  Gain=1/parGrid.WTstring.Rparallel_cable
  Transformer-complex : Subsystem
  WFG : Subsystem
  V_out : Outport
  I_out : Outport  Port=2
  wires:
  I_in/1 -> C_cable/2(I_in)
  Gain/1 -> Add1/2
  Add1/1 -> C_cable/1(It2)
  Transformer-complex/2(It2) -> Add1/1
  V_in/1 -> Cable line/1(V)
  Transformer-complex/1(It1) -> C_filter/2(It1)
  Cable line/1(I_cable) -> C_cable/3(I_cable), I_out/1
  C_filter/1(Vc_filter_qd) -> WFG/1(vcqd), Transformer-complex/1(Vc_filter_qd)
  WFG/1(ilqd) -> C_filter/1(IWF)
  C_cable/1(Vc_cable) -> Cable line/2(Vc_cable), V_out/1, Transformer-complex/2(Vc_cable_qd), Gain/1

== system wind1000_BUS_Original/Subsystem/Subsystem_1/Subsystem/String4/WT10 ==
  V_in : Inport
  I_in : Inport  Port=2
  Add1 : Sum  Inputs=+-
  C_cable : Subsystem
  C_filter : Subsystem
  Cable line : Subsystem
  Gain : Gain  Gain=1/parGrid.WTstring.Rparallel_cable
  Transformer-complex : Subsystem
  WFG : Subsystem
  V_out : Outport
  I_out : Outport  Port=2
  wires:
  C_cable/1(Vc_cable) -> Gain/1, Transformer-complex/2(Vc_cable_qd), V_out/1, Cable line/2(Vc_cable)
  WFG/1(ilqd) -> C_filter/1(IWF)
  C_filter/1(Vc_filter_qd) -> Transformer-complex/1(Vc_filter_qd), WFG/1(vcqd)
  Cable line/1(I_cable) -> I_out/1, C_cable/3(I_cable)
  Transformer-complex/1(It1) -> C_filter/2(It1)
  V_in/1 -> Cable line/1(V)
  Transformer-complex/2(It2) -> Add1/1
  Add1/1 -> C_cable/1(It2)
  Gain/1 -> Add1/2
  I_in/1 -> C_cable/2(I_in)

== system wind1000_BUS_Original/Subsystem/Subsystem_1/Subsystem/String4/WT2 ==
  V_in : Inport
  I_in : Inport  Port=2
  Add1 : Sum  Inputs=+-
  C_cable : Subsystem
  C_filter : Subsystem
  Cable line : Subsystem
  Gain : Gain  Gain=1/parGrid.WTstring.Rparallel_cable
  Transformer-complex : Subsystem
  WFG : Subsystem
  V_out : Outport
  I_out : Outport  Port=2
  wires:
  C_cable/1(Vc_cable) -> Gain/1, Transformer-complex/2(Vc_cable_qd), V_out/1, Cable line/2(Vc_cable)
  WFG/1(ilqd) -> C_filter/1(IWF)
  C_filter/1(Vc_filter_qd) -> Transformer-complex/1(Vc_filter_qd), WFG/1(vcqd)
  Cable line/1(I_cable) -> I_out/1, C_cable/3(I_cable)
  Transformer-complex/1(It1) -> C_filter/2(It1)
  V_in/1 -> Cable line/1(V)
  Transformer-complex/2(It2) -> Add1/1
  Add1/1 -> C_cable/1(It2)
  Gain/1 -> Add1/2
  I_in/1 -> C_cable/2(I_in)

== system wind1000_BUS_Original/Subsystem/Subsystem_1/Subsystem/String4/WT3 ==
  V_in : Inport
  I_in : Inport  Port=2
  Add1 : Sum  Inputs=+-
  C_cable : Subsystem
  C_filter : Subsystem
  Cable line : Subsystem
  Gain : Gain  Gain=1/parGrid.WTstring.Rparallel_cable
  Transformer-complex : Subsystem
  WFG : Subsystem
  V_out : Outport
  I_out : Outport  Port=2
  wires:
  I_in/1 -> C_cable/2(I_in)
  Gain/1 -> Add1/2
  Add1/1 -> C_cable/1(It2)
  Transformer-complex/2(It2) -> Add1/1
  V_in/1 -> Cable line/1(V)
  Transformer-complex/1(It1) -> C_filter/2(It1)
  Cable line/1(I_cable) -> C_cable/3(I_cable), I_out/1
  C_filter/1(Vc_filter_qd) -> Transformer-complex/1(Vc_filter_qd), WFG/1(vcqd)
  WFG/1(ilqd) -> C_filter/1(IWF)
  C_cable/1(Vc_cable) -> Cable line/2(Vc_cable), V_out/1, Transformer-complex/2(Vc_cable_qd), Gain/1

== system wind1000_BUS_Original/Subsystem/Subsystem_1/Subsystem/String4/WT4 ==
  V_in : Inport
  I_in : Inport  Port=2
  Add1 : Sum  Inputs=+-
  C_cable : Subsystem
  C_filter : Subsystem
  Cable line : Subsystem
  Gain : Gain  Gain=1/parGrid.WTstring.Rparallel_cable
  Transformer-complex : Subsystem
  WFG : Subsystem
  V_out : Outport
  I_out : Outport  Port=2
  wires:
  C_cable/1(Vc_cable) -> Gain/1, Transformer-complex/2(Vc_cable_qd), V_out/1, Cable line/2(Vc_cable)
  WFG/1(ilqd) -> C_filter/1(IWF)
  C_filter/1(Vc_filter_qd) -> Transformer-complex/1(Vc_filter_qd), WFG/1(vcqd)
  Cable line/1(I_cable) -> I_out/1, C_cable/3(I_cable)
  Transformer-complex/1(It1) -> C_filter/2(It1)
  V_in/1 -> Cable line/1(V)
  Transformer-complex/2(It2) -> Add1/1
  Add1/1 -> C_cable/1(It2)
  Gain/1 -> Add1/2
  I_in/1 -> C_cable/2(I_in)

== system wind1000_BUS_Original/Subsystem/Subsystem_1/Subsystem/String4/WT5 ==
  V_in : Inport
  I_in : Inport  Port=2
  Add1 : Sum  Inputs=+-
  C_cable : Subsystem
  C_filter : Subsystem
  Cable line : Subsystem
  Gain : Gain  Gain=1/parGrid.WTstring.Rparallel_cable
  Transformer-complex : Subsystem
  WFG : Subsystem
  V_out : Outport
  I_out : Outport  Port=2
  wires:
  I_in/1 -> C_cable/2(I_in)
  Gain/1 -> Add1/2
  Add1/1 -> C_cable/1(It2)
  Transformer-complex/2(It2) -> Add1/1
  V_in/1 -> Cable line/1(V)
  Transformer-complex/1(It1) -> C_filter/2(It1)
  Cable line/1(I_cable) -> C_cable/3(I_cable), I_out/1
  C_filter/1(Vc_filter_qd) -> Transformer-complex/1(Vc_filter_qd), WFG/1(vcqd)
  WFG/1(ilqd) -> C_filter/1(IWF)
  C_cable/1(Vc_cable) -> Cable line/2(Vc_cable), V_out/1, Transformer-complex/2(Vc_cable_qd), Gain/1

== system wind1000_BUS_Original/Subsystem/Subsystem_1/Subsystem/String4/WT6 ==
  V_in : Inport
  I_in : Inport  Port=2
  Add1 : Sum  Inputs=+-
  C_cable : Subsystem
  C_filter : Subsystem
  Cable line : Subsystem
  Gain : Gain  Gain=1/parGrid.WTstring.Rparallel_cable
  Transformer-complex : Subsystem
  WFG : Subsystem
  V_out : Outport
  I_out : Outport  Port=2
  wires:
  C_cable/1(Vc_cable) -> Gain/1, Transformer-complex/2(Vc_cable_qd), V_out/1, Cable line/2(Vc_cable)
  WFG/1(ilqd) -> C_filter/1(IWF)
  C_filter/1(Vc_filter_qd) -> Transformer-complex/1(Vc_filter_qd), WFG/1(vcqd)
  Cable line/1(I_cable) -> I_out/1, C_cable/3(I_cable)
  Transformer-complex/1(It1) -> C_filter/2(It1)
  V_in/1 -> Cable line/1(V)
  Transformer-complex/2(It2) -> Add1/1
  Add1/1 -> C_cable/1(It2)
  Gain/1 -> Add1/2
  I_in/1 -> C_cable/2(I_in)

== system wind1000_BUS_Original/Subsystem/Subsystem_1/Subsystem/String4/WT7 ==
  V_in : Inport
  I_in : Inport  Port=2
  Add1 : Sum  Inputs=+-
  C_cable : Subsystem
  C_filter : Subsystem
  Cable line : Subsystem
  Gain : Gain  Gain=1/parGrid.WTstring.Rparallel_cable
  Transformer-complex : Subsystem
  WFG : Subsystem
  V_out : Outport
  I_out : Outport  Port=2
  wires:
  I_in/1 -> C_cable/2(I_in)
  Gain/1 -> Add1/2
  Add1/1 -> C_cable/1(It2)
  Transformer-complex/2(It2) -> Add1/1
  V_in/1 -> Cable line/1(V)
  Transformer-complex/1(It1) -> C_filter/2(It1)
  Cable line/1(I_cable) -> C_cable/3(I_cable), I_out/1
  C_filter/1(Vc_filter_qd) -> Transformer-complex/1(Vc_filter_qd), WFG/1(vcqd)
  WFG/1(ilqd) -> C_filter/1(IWF)
  C_cable/1(Vc_cable) -> Cable line/2(Vc_cable), V_out/1, Transformer-complex/2(Vc_cable_qd), Gain/1

== system wind1000_BUS_Original/Subsystem/Subsystem_1/Subsystem/String4/WT8 ==
  V_in : Inport
  I_in : Inport  Port=2
  Add1 : Sum  Inputs=+-
  C_cable : Subsystem
  C_filter : Subsystem
  Cable line : Subsystem
  Gain : Gain  Gain=1/parGrid.WTstring.Rparallel_cable
  Transformer-complex : Subsystem
  WFG : Subsystem
  V_out : Outport
  I_out : Outport  Port=2
  wires:
  C_cable/1(Vc_cable) -> Gain/1, Transformer-complex/2(Vc_cable_qd), V_out/1, Cable line/2(Vc_cable)
  WFG/1(ilqd) -> C_filter/1(IWF)
  C_filter/1(Vc_filter_qd) -> Transformer-complex/1(Vc_filter_qd), WFG/1(vcqd)
  Cable line/1(I_cable) -> I_out/1, C_cable/3(I_cable)
  Transformer-complex/1(It1) -> C_filter/2(It1)
  V_in/1 -> Cable line/1(V)
  Transformer-complex/2(It2) -> Add1/1
  Add1/1 -> C_cable/1(It2)
  Gain/1 -> Add1/2
  I_in/1 -> C_cable/2(I_in)

== system wind1000_BUS_Original/Subsystem/Subsystem_1/Subsystem/String4/WT9 ==
  V_in : Inport
  I_in : Inport  Port=2
  Add1 : Sum  Inputs=+-
  C_cable : Subsystem
  C_filter : Subsystem
  Cable line : Subsystem
  Gain : Gain  Gain=1/parGrid.WTstring.Rparallel_cable
  Transformer-complex : Subsystem
  WFG : Subsystem
  V_out : Outport
  I_out : Outport  Port=2
  wires:
  I_in/1 -> C_cable/2(I_in)
  Gain/1 -> Add1/2
  Add1/1 -> C_cable/1(It2)
  Transformer-complex/2(It2) -> Add1/1
  V_in/1 -> Cable line/1(V)
  Transformer-complex/1(It1) -> C_filter/2(It1)
  Cable line/1(I_cable) -> C_cable/3(I_cable), I_out/1
  C_filter/1(Vc_filter_qd) -> Transformer-complex/1(Vc_filter_qd), WFG/1(vcqd)
  WFG/1(ilqd) -> C_filter/1(IWF)
  C_cable/1(Vc_cable) -> Cable line/2(Vc_cable), V_out/1, Transformer-complex/2(Vc_cable_qd), Gain/1

== system wind1000_BUS_Original/Subsystem/Subsystem_1/Subsystem/String5/WT1 ==
  V_in : Inport
  I_in : Inport  Port=2
  Add1 : Sum  Inputs=+-
  C_cable : Subsystem
  C_filter : Subsystem
  Cable line : Subsystem
  Gain : Gain  Gain=1/parGrid.WTstring.Rparallel_cable
  Transformer-complex : Subsystem
  WFG : Subsystem
  V_out : Outport
  I_out : Outport  Port=2
  wires:
  I_in/1 -> C_cable/2(I_in)
  Gain/1 -> Add1/2
  Add1/1 -> C_cable/1(It2)
  Transformer-complex/2(It2) -> Add1/1
  V_in/1 -> Cable line/1(V)
  Transformer-complex/1(It1) -> C_filter/2(It1)
  Cable line/1(I_cable) -> C_cable/3(I_cable), I_out/1
  C_filter/1(Vc_filter_qd) -> WFG/1(vcqd), Transformer-complex/1(Vc_filter_qd)
  WFG/1(ilqd) -> C_filter/1(IWF)
  C_cable/1(Vc_cable) -> Cable line/2(Vc_cable), V_out/1, Transformer-complex/2(Vc_cable_qd), Gain/1

== system wind1000_BUS_Original/Subsystem/Subsystem_1/Subsystem/String5/WT10 ==
  V_in : Inport
  I_in : Inport  Port=2
  Add1 : Sum  Inputs=+-
  C_cable : Subsystem
  C_filter : Subsystem
  Cable line : Subsystem
  Gain : Gain  Gain=1/parGrid.WTstring.Rparallel_cable
  Transformer-complex : Subsystem
  WFG : Subsystem
  V_out : Outport
  I_out : Outport  Port=2
  wires:
  C_cable/1(Vc_cable) -> Gain/1, Transformer-complex/2(Vc_cable_qd), V_out/1, Cable line/2(Vc_cable)
  WFG/1(ilqd) -> C_filter/1(IWF)
  C_filter/1(Vc_filter_qd) -> Transformer-complex/1(Vc_filter_qd), WFG/1(vcqd)
  Cable line/1(I_cable) -> I_out/1, C_cable/3(I_cable)
  Transformer-complex/1(It1) -> C_filter/2(It1)
  V_in/1 -> Cable line/1(V)
  Transformer-complex/2(It2) -> Add1/1
  Add1/1 -> C_cable/1(It2)
  Gain/1 -> Add1/2
  I_in/1 -> C_cable/2(I_in)

== system wind1000_BUS_Original/Subsystem/Subsystem_1/Subsystem/String5/WT2 ==
  V_in : Inport
  I_in : Inport  Port=2
  Add1 : Sum  Inputs=+-
  C_cable : Subsystem
  C_filter : Subsystem
  Cable line : Subsystem
  Gain : Gain  Gain=1/parGrid.WTstring.Rparallel_cable
  Transformer-complex : Subsystem
  WFG : Subsystem
  V_out : Outport
  I_out : Outport  Port=2
  wires:
  C_cable/1(Vc_cable) -> Gain/1, Transformer-complex/2(Vc_cable_qd), V_out/1, Cable line/2(Vc_cable)
  WFG/1(ilqd) -> C_filter/1(IWF)
  C_filter/1(Vc_filter_qd) -> Transformer-complex/1(Vc_filter_qd), WFG/1(vcqd)
  Cable line/1(I_cable) -> I_out/1, C_cable/3(I_cable)
  Transformer-complex/1(It1) -> C_filter/2(It1)
  V_in/1 -> Cable line/1(V)
  Transformer-complex/2(It2) -> Add1/1
  Add1/1 -> C_cable/1(It2)
  Gain/1 -> Add1/2
  I_in/1 -> C_cable/2(I_in)

== system wind1000_BUS_Original/Subsystem/Subsystem_1/Subsystem/String5/WT3 ==
  V_in : Inport
  I_in : Inport  Port=2
  Add1 : Sum  Inputs=+-
  C_cable : Subsystem
  C_filter : Subsystem
  Cable line : Subsystem
  Gain : Gain  Gain=1/parGrid.WTstring.Rparallel_cable
  Transformer-complex : Subsystem
  WFG : Subsystem
  V_out : Outport
  I_out : Outport  Port=2
  wires:
  I_in/1 -> C_cable/2(I_in)
  Gain/1 -> Add1/2
  Add1/1 -> C_cable/1(It2)
  Transformer-complex/2(It2) -> Add1/1
  V_in/1 -> Cable line/1(V)
  Transformer-complex/1(It1) -> C_filter/2(It1)
  Cable line/1(I_cable) -> C_cable/3(I_cable), I_out/1
  C_filter/1(Vc_filter_qd) -> Transformer-complex/1(Vc_filter_qd), WFG/1(vcqd)
  WFG/1(ilqd) -> C_filter/1(IWF)
  C_cable/1(Vc_cable) -> Cable line/2(Vc_cable), V_out/1, Transformer-complex/2(Vc_cable_qd), Gain/1

== system wind1000_BUS_Original/Subsystem/Subsystem_1/Subsystem/String5/WT4 ==
  V_in : Inport
  I_in : Inport  Port=2
  Add1 : Sum  Inputs=+-
  C_cable : Subsystem
  C_filter : Subsystem
  Cable line : Subsystem
  Gain : Gain  Gain=1/parGrid.WTstring.Rparallel_cable
  Transformer-complex : Subsystem
  WFG : Subsystem
  V_out : Outport
  I_out : Outport  Port=2
  wires:
  C_cable/1(Vc_cable) -> Gain/1, Transformer-complex/2(Vc_cable_qd), V_out/1, Cable line/2(Vc_cable)
  WFG/1(ilqd) -> C_filter/1(IWF)
  C_filter/1(Vc_filter_qd) -> Transformer-complex/1(Vc_filter_qd), WFG/1(vcqd)
  Cable line/1(I_cable) -> I_out/1, C_cable/3(I_cable)
  Transformer-complex/1(It1) -> C_filter/2(It1)
  V_in/1 -> Cable line/1(V)
  Transformer-complex/2(It2) -> Add1/1
  Add1/1 -> C_cable/1(It2)
  Gain/1 -> Add1/2
  I_in/1 -> C_cable/2(I_in)

== system wind1000_BUS_Original/Subsystem/Subsystem_1/Subsystem/String5/WT5 ==
  V_in : Inport
  I_in : Inport  Port=2
  Add1 : Sum  Inputs=+-
  C_cable : Subsystem
  C_filter : Subsystem
  Cable line : Subsystem
  Gain : Gain  Gain=1/parGrid.WTstring.Rparallel_cable
  Transformer-complex : Subsystem
  WFG : Subsystem
  V_out : Outport
  I_out : Outport  Port=2
  wires:
  I_in/1 -> C_cable/2(I_in)
  Gain/1 -> Add1/2
  Add1/1 -> C_cable/1(It2)
  Transformer-complex/2(It2) -> Add1/1
  V_in/1 -> Cable line/1(V)
  Transformer-complex/1(It1) -> C_filter/2(It1)
  Cable line/1(I_cable) -> C_cable/3(I_cable), I_out/1
  C_filter/1(Vc_filter_qd) -> Transformer-complex/1(Vc_filter_qd), WFG/1(vcqd)
  WFG/1(ilqd) -> C_filter/1(IWF)
  C_cable/1(Vc_cable) -> Cable line/2(Vc_cable), V_out/1, Transformer-complex/2(Vc_cable_qd), Gain/1
